simulink model "V2x_TC_IntelligentTrafficSigns_cs"  (Simulink 7.6)
settings: Solver=FixedStepDiscrete  SolverMode=SingleTasking  StartTime=0.0  StopTime=66  FixedStep=1/100  RelTol=1e-3
model workspace (MAT data in the model file):
  mbox_table : struct (value not decoded)
PreLoadFcn:
  | check_NUTS_defined
  | 
  | assignin('base',['model_closed_' bdroot],false);addpath('../includes_V2x');
InitFcn:
  | PreScanExperimentInitFcn(bdroot)
StopFcn:
  | PreScanExperimentStopFcn(bdroot);
  | 
undefined symbols (not defined in the file or in its folder): acceleration, actorID, actorType, DiscIntNeverNeededParam, GPS_alt, GPS_lat, GPS_long, lightBarInUse, messageID, Newton, publicActorType, Raphson, rotZ, sigbuilder_block, sireneInUse, trafficLightPriority, turnAdvice, velocity, x_ini_VRML, y_ini_VRML, yawRate, z_ini_VRML
libraries: simulink x111, PreScanSimulinkLibrary x7, simulink_extras x4, PreScanUsefulBlocks x3
(part 1 of 16)
CloseFcn:
  | evalin('base',['clear model_closed_' bdroot]);
  | 
  | try
  |     close (V2x_TC_GUI)
  | end
  | 
  | try
  |     close (V2X_PlotSpeed)
  | end
settings Model: Creator=PRESCAN  LastModifiedBy=buildbot
settings OptimizationCC: InitFltsAndDblsToZero=on
settings DebuggingCC: SaveWithParameterizedLinksMsg=none  SignalResolutionControl=TryResolveAllWithWarning  MaxConsecutiveZCsMsg=warning  MultiTaskDSMMsg=warning  MultiTaskCondExecSysMsg=none
settings SFSimCC: SimParseCustomCode=off
settings RTWCC: IncludeERTFirstTime=on
settings GRTTargetCC: TargetFcnLib=ansi_tfl_tmw.mat

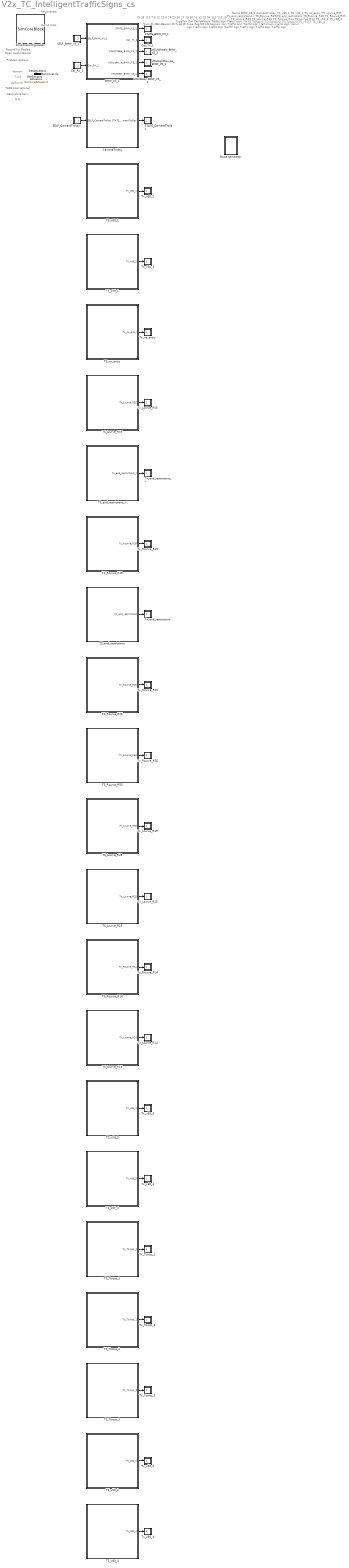
== system V2x_TC_IntelligentTrafficSigns_cs ==
  note: OBU-Beacon ID
  note: Demo Help
  note: Regenerate
  note: Record for Replay
  note: Open Useful Blocks
  note: PreScan Options
  note: Version:
  note: 7.4.0
  note: Author(s):
  note: TASS International
  note: Descriptive text:
  note: N.A.
  note: ID
      |
      | 28
      | 113
      | 7
      | 6
      | 22
      | 23
      | 8
      | 24
      | 25
      | 26
      | 27
      | 29
      | 30
      | 31
      | 32
      | 33
      | 34
      | 111
      | 112
      | 19
      | 20
      | 38
  note: OBU-Beacon ID
  note: TypeID
      |
      | 1
      | 5
      | 14
      | 14
      | 14
      | 14
      | 14
      | 14
      | 14
      | 14
      | 14
      | 14
      | 14
      | 14
      | 14
      | 14
      | 14
      | 14
      | 14
      | 14
      | 14
      | 14
  note: TypeTag
      |
      | Car
      | Cal element
      | Traffic sign
      | Traffic sign
      | Traffic sign
      | Traffic sign
      | Traffic sign
      | Traffic sign
      | Traffic sign
      | Traffic sign
      | Traffic sign
      | Traffic sign
      | Traffic sign
      | Traffic sign
      | Traffic sign
      | Traffic sign
      | Traffic sign
      | Traffic sign
      | Traffic sign
      | Traffic sign
      | Traffic sign
      | Traffic sign
  note: SD
      |
      | Y
      | Y
      | N
      | N
      | N
      | N
      | N
      | N
      | N
      | N
      | N
      | N
      | N
      | N
      | N
      | N
      | N
      | N
      | N
      | N
      | N
      | N
  note: CD
      |
      | N
      | N
      | N
      | N
      | N
      | N
      | N
      | N
      | N
      | N
      | N
      | N
      | N
      | N
      | N
      | N
      | N
      | N
      | N
      | N
      | N
      | N
  note: Name
      |
      | BMW_X5_1
      | CameraTrolley
      | TS_v80_1
      | TS_v50_1
      | TS_no_entry
      | TS_Lcurve_R55
      | TS_end_restrictions_r
      | TS_Rcurve_R39
      | TS_end_restrictions
      | TS_Rcurve_R25
      | TS_Rcurve_R55
      | TS_Lcurve_R39
      | TS_Lcurve_R25
      | TS_Rcurve_R14
      | TS_Lcurve_R14
      | TS_v50_3
      | TS_v80_3
      | TS_Tcross_1
      | TS_Tcross_2
      | TS_Tcross_3
      | TS_v50_2
      | TS_v80_4
  note: Num ID OBU-Beacon ID Type ID Type Tag SD CD Name
  Actuators : DataStoreMemory  Tag=Actuators  DataStoreName=SimCoreActuators  InitialValue=zeros(4563,1)
      settings: DeleteFcn=PreScanBlockDeleteFcn;
  BMW_X5_1 : Subsystem  Tag=WorldObjectSubSystem  InitFcn=UniqueId = '93';
      mask Display: image(imread('BMW_X5.jpg'));color('white');text(0,0,' ID=28\n')
  CameraTrolley : Subsystem  Tag=WorldObjectSubSystem  InitFcn=UniqueId = '98';
      mask Display: image(imread('Sphere.jpg'));color('white');text(0,0,' ID=113\n')
  Car_Rx_1 : Subsystem  Tag=AntennaR  Priority=3  mask(PreScan Source Block: Antenna Receiver){Channel=1, NrEntitiesOut=5, PortName=Car_Rx_1, PortIndex=8, PortWidth=1140, TimeStep=1/100}
      mask prompts: Channel "User Specified: Channel# [-]"; NrEntitiesOut "User Specified: # of Visible Actors"; PortName "Auto Assigned: Port Name [-]"; PortIndex "Auto Assigned: Index [-]"; PortWidth "Auto Assigned: Port Dimensions [-]"; TimeStep "Auto Assigned: Upload Period [s]"
      mask help:
      | Using the Antenna Receiver (RX) and Transmitter (TX) information between actors can be exchanged. This is done over a channel number to be specified by the user.
      |
      | The Antenna Reciever receives actor information and user-definable signals.
      |
      | For more information, please click Help.
      settings: DeleteFcn=PreScanBlockDeleteFcn;
      mask Help: eval('openHelp(424)')
      mask Display: image(imread('Rx.png'))
  Car_Tx_1 : Subsystem  Tag=AntennaT  Priority=0  mask(PreScan Sink Block: AntennaT){Channel=1, PortName=Car_Tx_1, PortIndex=15, PortWidth=205, TimeStep=1/100}
      mask prompts: Channel "User Specified: Channel# [-]"; PortName "Auto Assigned: Port Name [-]"; PortIndex "Auto Assigned: Index [-]"; PortWidth "Auto Assigned: Port Dimensions [-]"; TimeStep "Auto Assigned: Upload Period [s]"
      mask help:
      | Using the Antenna Receiver (RX) and Transmitter (TX) information between actors can be exchanged. This is done over a channel number to be specified by the user.
      |
      | The Antenna Transmitter contains the following, user-definable signals:
      |
      | - Warning Signal ID	[-]
      | - Maneuvre ID		[-]
      | - Vehicle Type 		[-]
      | - Braking Capacity	[-]
      | - Vehicle Size		[-]
      | - Spares (1:10)		[-]
      |
      | For more information, please click Help.
      settings: DeleteFcn=PreScanBlockDeleteFcn;
      mask Help: eval('openHelp(424)')
      mask Display: image(imread('Tx.png'))
  GetSensors : Subsystem  Tag=GetSensors  Priority=2  mask(PreScan Simulation Core Block){NrOfSensors=1156, TimeStep=1/100}
      mask prompts: NrOfSensors "Number of sensor values"; TimeStep "Timestep [s]"
      mask help:
      | Please do not remove this block from the PreScan compilation sheet,
      | as it contains essential configuration parameters for a PreScan simulation.
      settings: DeleteFcn=PreScanBlockDeleteFcn;
  Noise generator : Subsystem
  SELF_BMW_X5_1 : Subsystem  Tag=SELF  Priority=3  mask(PreScan Source Block: SELF){PortName=SELF_BMW_X5_1, PortIndex=0, PortWidth=8, TimeStep=1/100}
      mask prompts: PortName "Auto-Assigned: Port Name [-]"; PortIndex "Auto-Assigned: Port Index [-]"; PortWidth "Auto-Assigned: Port Dimensions"; TimeStep "Auto-Assigned: Upload Period [s]"
      mask help:
      | The SELF port shows (state) information of the actor itself. The outputs are position in the experiment's axis system and the GPS coordinates, and the object's absolute velocity and compass heading.
      |
      | For more information, please click Help.
      settings: DeleteFcn=PreScanBlockDeleteFcn;
      mask Help: eval('openHelp(462)')
      mask Display: image(imread('GPS.png'))
  SELF_CameraTrolley : Subsystem  Tag=SELF  Priority=3  mask(PreScan Source Block: SELF){PortName=SELF_CameraTrolley, PortIndex=1148, PortWidth=8, TimeStep=1/100}
      mask prompts: PortName "Auto-Assigned: Port Name [-]"; PortIndex "Auto-Assigned: Port Index [-]"; PortWidth "Auto-Assigned: Port Dimensions"; TimeStep "Auto-Assigned: Upload Period [s]"
      mask help:
      | The SELF port shows (state) information of the actor itself. The outputs are position in the experiment's axis system and the GPS coordinates, and the object's absolute velocity and compass heading.
      |
      | For more information, please click Help.
      settings: DeleteFcn=PreScanBlockDeleteFcn;
      mask Help: eval('openHelp(462)')
      mask Display: image(imread('GPS.png'))
  STATE_BMW_X5_1 : Subsystem  Tag=State  Priority=0  mask(PreScan Sink Block: State){x0=80, y0=1.75, z0=0.69, Roll0=0, Pitch0=0, Yaw0=179.999588, TimeStep=1/100}
      mask prompts: x0 "Ini x-position VEHICLE wrt GLOBAL axis system [m]"; y0 "Ini y-position VEHICLE wrt GLOBAL axis system [m]"; z0 "Ini z-position VEHICLE wrt GLOBAL axis system [m]"; Roll0 "Ini roll-angle BODY wrt VEHICLE axis system [deg]"; Pitch0 "Ini pitch-angle BODY wrt VEHICLE axis system [deg]"; Yaw0 "Ini yaw-angle VEHICLE wrt GLOBAL axis system [deg]"; TimeStep "Time Step [s]"
      mask help:
      | The Actor State contains a vector signal of size (9x1), containing the following signals:
      |
      | -  Position                  (x,y,z)                                     [m]
      | -  Velocity                  (vx,vy,vz)                               [m/s]
      | -  Angles                    (Roll, Pitch, Yaw)                   [rad]
      |
      | The Actor state is defined in the global axis system.
      | The state is superponed on the Initial position defined in the GUI.
      |
      | For more information, please click Help.
      settings: DeleteFcn=PreScanBlockDeleteFcn;
      mask Help: eval('openHelp(222)')
      mask Display: image(imread('State.png'))
  STATE_CameraTrolley : Subsystem  Tag=State  Priority=0  mask(PreScan Sink Block: State){x0=80, y0=1.75, z0=0.69, Roll0=0, Pitch0=0, Yaw0=179.9996, TimeStep=1/100}
      mask prompts: x0 "Ini x-position VEHICLE wrt GLOBAL axis system [m]"; y0 "Ini y-position VEHICLE wrt GLOBAL axis system [m]"; z0 "Ini z-position VEHICLE wrt GLOBAL axis system [m]"; Roll0 "Ini roll-angle BODY wrt VEHICLE axis system [deg]"; Pitch0 "Ini pitch-angle BODY wrt VEHICLE axis system [deg]"; Yaw0 "Ini yaw-angle VEHICLE wrt GLOBAL axis system [deg]"; TimeStep "Time Step [s]"
      mask help:
      | The Actor State contains a vector signal of size (9x1), containing the following signals:
      |
      | -  Position                  (x,y,z)                                     [m]
      | -  Velocity                  (vx,vy,vz)                               [m/s]
      | -  Angles                    (Roll, Pitch, Yaw)                   [rad]
      |
      | The Actor state is defined in the global axis system.
      | The state is superponed on the Initial position defined in the GUI.
      |
      | For more information, please click Help.
      settings: DeleteFcn=PreScanBlockDeleteFcn;
      mask Help: eval('openHelp(222)')
      mask Display: image(imread('State.png'))
  SetActuators : Subsystem  Tag=SetActuators  Priority=1  mask(PreScan Simulation Core Block){TimeStep=1/100, NrOfActuators=4563}
      mask prompts: TimeStep "Timestep [s]"; NrOfActuators "Number of actuator values"
      mask help:
      | Please do not remove this block from the PreScan compilation sheet,
      | as it contains essential configuration parameters for a PreScan simulation.
      settings: DeleteFcn=PreScanBlockDeleteFcn;
  SimCoreConfiguration : M-S-Function  Tag=SimCoreConfiguration  Description=AE5066EEE462D865D5F999FC1C49C4DE43F133A736DE7B82F5B9C11F164EF63C  FunctionName=SimCoreBlock  Parameters=ExperimentXML PortsXML SimulationCore HostName CheckStart EnableLog RfR EnableGPUAccel ShowComputationTime  mask(PreScan Simulation Core Configurator){SimulationCore=NUTS, HostName=0, CheckStart=0, prescanversion='7.4.0', EnableLog=off, RfR=0, EnableGPUAccel=off, ShowComputationTime=off}
      mask prompts: SimulationCore "Simulation Core"; HostName "Simulation Host"; CheckStart "Show START Dialog"; prescanversion "PreScan Version"; EnableLog "Create TA Log"; RfR "Record for Replay"; EnableGPUAccel "Enable GPU Acceleration"; ShowComputationTime "Show ComputationTime Scope"
      mask help:
      | Please do not remove this block from the PreScan compilation sheet,
      | as it contains essential configuration parameter for a PreScan simulation.
      mask init:
      | model = bdroot;
      | ExperimentXML = [model(1:end-3) '.pex'];
      | PortsXML = [model '_ports.pcd'];
      settings: DeleteFcn=PreScanBlockDeleteFcn;  ModelCloseFcn=assignin('base',['model_closed_' bdroot],true);
      mask Help: eval('openHelp(812)')
      mask Display: image(imread('prescanlogo.ico'))
  SimCoreInfo : Subsystem  Tag=SimCoreInfo
  TS_Lcurve_R14 : Subsystem  Tag=WorldObjectSubSystem  InitFcn=UniqueId = '76';
      mask Display: image(imread('TrafficSignPoleNL.jpg'));color('white');text(0,0,' ID=32\n')
  TS_Lcurve_R25 : Subsystem  Tag=WorldObjectSubSystem  InitFcn=UniqueId = '72';
      mask Display: image(imread('TrafficSignPoleNL.jpg'));color('white');text(0,0,' ID=30\n')
  TS_Lcurve_R39 : Subsystem  Tag=WorldObjectSubSystem  InitFcn=UniqueId = '70';
      mask Display: image(imread('TrafficSignPoleNL.jpg'));color('white');text(0,0,' ID=29\n')
  TS_Lcurve_R55 : Subsystem  Tag=WorldObjectSubSystem  InitFcn=UniqueId = '58';
      mask Display: image(imread('TrafficSignPoleNL.jpg'));color('white');text(0,0,' ID=23\n')
  TS_Rcurve_R14 : Subsystem  Tag=WorldObjectSubSystem  InitFcn=UniqueId = '74';
      mask Display: image(imread('TrafficSignPoleNL.jpg'));color('white');text(0,0,' ID=31\n')
  TS_Rcurve_R25 : Subsystem  Tag=WorldObjectSubSystem  InitFcn=UniqueId = '66';
      mask Display: image(imread('TrafficSignPoleNL.jpg'));color('white');text(0,0,' ID=26\n')
  TS_Rcurve_R39 : Subsystem  Tag=WorldObjectSubSystem  InitFcn=UniqueId = '62';
      mask Display: image(imread('TrafficSignPoleNL.jpg'));color('white');text(0,0,' ID=24\n')
  TS_Rcurve_R55 : Subsystem  Tag=WorldObjectSubSystem  InitFcn=UniqueId = '68';
      mask Display: image(imread('TrafficSignPoleNL.jpg'));color('white');text(0,0,' ID=27\n')
  TS_Tcross_1 : Subsystem  Tag=WorldObjectSubSystem  InitFcn=UniqueId = '82';
      mask Display: image(imread('TrafficSignPoleNL.jpg'));color('white');text(0,0,' ID=111\n')
  TS_Tcross_2 : Subsystem  Tag=WorldObjectSubSystem  InitFcn=UniqueId = '84';
      mask Display: image(imread('TrafficSignPoleNL.jpg'));color('white');text(0,0,' ID=112\n')
  TS_Tcross_3 : Subsystem  Tag=WorldObjectSubSystem  InitFcn=UniqueId = '120';
      mask Display: image(imread('TrafficSignPoleNL.jpg'));color('white');text(0,0,' ID=19\n')
  TS_end_restrictions : Subsystem  Tag=WorldObjectSubSystem  InitFcn=UniqueId = '64';
      mask Display: image(imread('TrafficSignPoleNL.jpg'));color('white');text(0,0,' ID=25\n')
  TS_end_restrictions_r : Subsystem  Tag=WorldObjectSubSystem  InitFcn=UniqueId = '60';
      mask Display: image(imread('TrafficSignPoleNL.jpg'));color('white');text(0,0,' ID=8\n')
  TS_no_entry : Subsystem  Tag=WorldObjectSubSystem  InitFcn=UniqueId = '56';
      mask Display: image(imread('TrafficSignPoleNL.jpg'));color('white');text(0,0,' ID=22\n')
  TS_v50_1 : Subsystem  Tag=WorldObjectSubSystem  InitFcn=UniqueId = '54';
      mask Display: image(imread('TrafficSignPoleNL.jpg'));color('white');text(0,0,' ID=6\n')
  TS_v50_2 : Subsystem  Tag=WorldObjectSubSystem  InitFcn=UniqueId = '122';
      mask Display: image(imread('TrafficSignPoleNL.jpg'));color('white');text(0,0,' ID=20\n')
  TS_v50_3 : Subsystem  Tag=WorldObjectSubSystem  InitFcn=UniqueId = '78';
      mask Display: image(imread('TrafficSignPoleNL.jpg'));color('white');text(0,0,' ID=33\n')
  TS_v80_1 : Subsystem  Tag=WorldObjectSubSystem  InitFcn=UniqueId = '52';
      mask Display: image(imread('TrafficSignPoleNL.jpg'));color('white');text(0,0,' ID=7\n')
  TS_v80_3 : Subsystem  Tag=WorldObjectSubSystem  InitFcn=UniqueId = '80';
      mask Display: image(imread('TrafficSignPoleNL.jpg'));color('white');text(0,0,' ID=34\n')
  TS_v80_4 : Subsystem  Tag=WorldObjectSubSystem  InitFcn=UniqueId = '125';
      mask Display: image(imread('TrafficSignPoleNL.jpg'));color('white');text(0,0,' ID=38\n')
  Tx_Lcurve_R14 : Subsystem  Tag=AntennaT  Priority=0  mask(PreScan Sink Block: AntennaT){Channel=1, PortName=Tx_Lcurve_R14, PortIndex=2923, PortWidth=205, TimeStep=1/100}
      mask prompts: Channel "User Specified: Channel# [-]"; PortName "Auto Assigned: Port Name [-]"; PortIndex "Auto Assigned: Index [-]"; PortWidth "Auto Assigned: Port Dimensions [-]"; TimeStep "Auto Assigned: Upload Period [s]"
      mask help:
      | Using the Antenna Receiver (RX) and Transmitter (TX) information between actors can be exchanged. This is done over a channel number to be specified by the user.
      |
      | The Antenna Transmitter contains the following, user-definable signals:
      |
      | - Warning Signal ID	[-]
      | - Maneuvre ID		[-]
      | - Vehicle Type 		[-]
      | - Braking Capacity	[-]
      | - Vehicle Size		[-]
      | - Spares (1:10)		[-]
      |
      | For more information, please click Help.
      settings: DeleteFcn=PreScanBlockDeleteFcn;
      mask Help: eval('openHelp(424)')
      mask Display: image(imread('Tx.png'))
  Tx_Lcurve_R25 : Subsystem  Tag=AntennaT  Priority=0  mask(PreScan Sink Block: AntennaT){Channel=1, PortName=Tx_Lcurve_R25, PortIndex=2513, PortWidth=205, TimeStep=1/100}
      mask prompts: Channel "User Specified: Channel# [-]"; PortName "Auto Assigned: Port Name [-]"; PortIndex "Auto Assigned: Index [-]"; PortWidth "Auto Assigned: Port Dimensions [-]"; TimeStep "Auto Assigned: Upload Period [s]"
      mask help:
      | Using the Antenna Receiver (RX) and Transmitter (TX) information between actors can be exchanged. This is done over a channel number to be specified by the user.
      |
      | The Antenna Transmitter contains the following, user-definable signals:
      |
      | - Warning Signal ID	[-]
      | - Maneuvre ID		[-]
      | - Vehicle Type 		[-]
      | - Braking Capacity	[-]
      | - Vehicle Size		[-]
      | - Spares (1:10)		[-]
      |
      | For more information, please click Help.
      settings: DeleteFcn=PreScanBlockDeleteFcn;
      mask Help: eval('openHelp(424)')
      mask Display: image(imread('Tx.png'))
  Tx_Lcurve_R39 : Subsystem  Tag=AntennaT  Priority=0  mask(PreScan Sink Block: AntennaT){Channel=1, PortName=Tx_Lcurve_R39, PortIndex=2308, PortWidth=205, TimeStep=1/100}
      mask prompts: Channel "User Specified: Channel# [-]"; PortName "Auto Assigned: Port Name [-]"; PortIndex "Auto Assigned: Index [-]"; PortWidth "Auto Assigned: Port Dimensions [-]"; TimeStep "Auto Assigned: Upload Period [s]"
      mask help:
      | Using the Antenna Receiver (RX) and Transmitter (TX) information between actors can be exchanged. This is done over a channel number to be specified by the user.
      |
      | The Antenna Transmitter contains the following, user-definable signals:
      |
      | - Warning Signal ID	[-]
      | - Maneuvre ID		[-]
      | - Vehicle Type 		[-]
      | - Braking Capacity	[-]
      | - Vehicle Size		[-]
      | - Spares (1:10)		[-]
      |
      | For more information, please click Help.
      settings: DeleteFcn=PreScanBlockDeleteFcn;
      mask Help: eval('openHelp(424)')
      mask Display: image(imread('Tx.png'))
  Tx_Lcurve_R55 : Subsystem  Tag=AntennaT  Priority=0  mask(PreScan Sink Block: AntennaT){Channel=1, PortName=Tx_Lcurve_R55, PortIndex=1078, PortWidth=205, TimeStep=1/100}
      mask prompts: Channel "User Specified: Channel# [-]"; PortName "Auto Assigned: Port Name [-]"; PortIndex "Auto Assigned: Index [-]"; PortWidth "Auto Assigned: Port Dimensions [-]"; TimeStep "Auto Assigned: Upload Period [s]"
      mask help:
      | Using the Antenna Receiver (RX) and Transmitter (TX) information between actors can be exchanged. This is done over a channel number to be specified by the user.
      |
      | The Antenna Transmitter contains the following, user-definable signals:
      |
      | - Warning Signal ID	[-]
      | - Maneuvre ID		[-]
      | - Vehicle Type 		[-]
      | - Braking Capacity	[-]
      | - Vehicle Size		[-]
      | - Spares (1:10)		[-]
      |
      | For more information, please click Help.
      settings: DeleteFcn=PreScanBlockDeleteFcn;
      mask Help: eval('openHelp(424)')
      mask Display: image(imread('Tx.png'))
  Tx_Rcurve_R14 : Subsystem  Tag=AntennaT  Priority=0  mask(PreScan Sink Block: AntennaT){Channel=1, PortName=Tx_Rcurve_R14, PortIndex=2718, PortWidth=205, TimeStep=1/100}
      mask prompts: Channel "User Specified: Channel# [-]"; PortName "Auto Assigned: Port Name [-]"; PortIndex "Auto Assigned: Index [-]"; PortWidth "Auto Assigned: Port Dimensions [-]"; TimeStep "Auto Assigned: Upload Period [s]"
      mask help:
      | Using the Antenna Receiver (RX) and Transmitter (TX) information between actors can be exchanged. This is done over a channel number to be specified by the user.
      |
      | The Antenna Transmitter contains the following, user-definable signals:
      |
      | - Warning Signal ID	[-]
      | - Maneuvre ID		[-]
      | - Vehicle Type 		[-]
      | - Braking Capacity	[-]
      | - Vehicle Size		[-]
      | - Spares (1:10)		[-]
      |
      | For more information, please click Help.
      settings: DeleteFcn=PreScanBlockDeleteFcn;
      mask Help: eval('openHelp(424)')
      mask Display: image(imread('Tx.png'))
  Tx_Rcurve_R25 : Subsystem  Tag=AntennaT  Priority=0  mask(PreScan Sink Block: AntennaT){Channel=1, PortName=Tx_Rcurve_R25, PortIndex=1898, PortWidth=205, TimeStep=1/100}
      mask prompts: Channel "User Specified: Channel# [-]"; PortName "Auto Assigned: Port Name [-]"; PortIndex "Auto Assigned: Index [-]"; PortWidth "Auto Assigned: Port Dimensions [-]"; TimeStep "Auto Assigned: Upload Period [s]"
      mask help:
      | Using the Antenna Receiver (RX) and Transmitter (TX) information between actors can be exchanged. This is done over a channel number to be specified by the user.
      |
      | The Antenna Transmitter contains the following, user-definable signals:
      |
      | - Warning Signal ID	[-]
      | - Maneuvre ID		[-]
      | - Vehicle Type 		[-]
      | - Braking Capacity	[-]
      | - Vehicle Size		[-]
      | - Spares (1:10)		[-]
      |
      | For more information, please click Help.
      settings: DeleteFcn=PreScanBlockDeleteFcn;
      mask Help: eval('openHelp(424)')
      mask Display: image(imread('Tx.png'))
  Tx_Rcurve_R39 : Subsystem  Tag=AntennaT  Priority=0  mask(PreScan Sink Block: AntennaT){Channel=1, PortName=Tx_Rcurve_R39, PortIndex=1488, PortWidth=205, TimeStep=1/100}
      mask prompts: Channel "User Specified: Channel# [-]"; PortName "Auto Assigned: Port Name [-]"; PortIndex "Auto Assigned: Index [-]"; PortWidth "Auto Assigned: Port Dimensions [-]"; TimeStep "Auto Assigned: Upload Period [s]"
      mask help:
      | Using the Antenna Receiver (RX) and Transmitter (TX) information between actors can be exchanged. This is done over a channel number to be specified by the user.
      |
      | The Antenna Transmitter contains the following, user-definable signals:
      |
      | - Warning Signal ID	[-]
      | - Maneuvre ID		[-]
      | - Vehicle Type 		[-]
      | - Braking Capacity	[-]
      | - Vehicle Size		[-]
      | - Spares (1:10)		[-]
      |
      | For more information, please click Help.
      settings: DeleteFcn=PreScanBlockDeleteFcn;
      mask Help: eval('openHelp(424)')
      mask Display: image(imread('Tx.png'))
  Tx_Rcurve_R55 : Subsystem  Tag=AntennaT  Priority=0  mask(PreScan Sink Block: AntennaT){Channel=1, PortName=Tx_Rcurve_R55, PortIndex=2103, PortWidth=205, TimeStep=1/100}
      mask prompts: Channel "User Specified: Channel# [-]"; PortName "Auto Assigned: Port Name [-]"; PortIndex "Auto Assigned: Index [-]"; PortWidth "Auto Assigned: Port Dimensions [-]"; TimeStep "Auto Assigned: Upload Period [s]"
      mask help:
      | Using the Antenna Receiver (RX) and Transmitter (TX) information between actors can be exchanged. This is done over a channel number to be specified by the user.
      |
      | The Antenna Transmitter contains the following, user-definable signals:
      |
      | - Warning Signal ID	[-]
      | - Maneuvre ID		[-]
      | - Vehicle Type 		[-]
      | - Braking Capacity	[-]
      | - Vehicle Size		[-]
      | - Spares (1:10)		[-]
      |
      | For more information, please click Help.
      settings: DeleteFcn=PreScanBlockDeleteFcn;
      mask Help: eval('openHelp(424)')
      mask Display: image(imread('Tx.png'))
  Tx_Tcross_1 : Subsystem  Tag=AntennaT  Priority=0  mask(PreScan Sink Block: AntennaT){Channel=1, PortName=Tx_Tcross_1, PortIndex=3538, PortWidth=205, TimeStep=1/100}
      mask prompts: Channel "User Specified: Channel# [-]"; PortName "Auto Assigned: Port Name [-]"; PortIndex "Auto Assigned: Index [-]"; PortWidth "Auto Assigned: Port Dimensions [-]"; TimeStep "Auto Assigned: Upload Period [s]"
      mask help:
      | Using the Antenna Receiver (RX) and Transmitter (TX) information between actors can be exchanged. This is done over a channel number to be specified by the user.
      |
      | The Antenna Transmitter contains the following, user-definable signals:
      |
      | - Warning Signal ID	[-]
      | - Maneuvre ID		[-]
      | - Vehicle Type 		[-]
      | - Braking Capacity	[-]
      | - Vehicle Size		[-]
      | - Spares (1:10)		[-]
      |
      | For more information, please click Help.
      settings: DeleteFcn=PreScanBlockDeleteFcn;
      mask Help: eval('openHelp(424)')
      mask Display: image(imread('Tx.png'))
  Tx_Tcross_2 : Subsystem  Tag=AntennaT  Priority=0  mask(PreScan Sink Block: AntennaT){Channel=1, PortName=Tx_Tcross_2, PortIndex=3743, PortWidth=205, TimeStep=1/100}
      mask prompts: Channel "User Specified: Channel# [-]"; PortName "Auto Assigned: Port Name [-]"; PortIndex "Auto Assigned: Index [-]"; PortWidth "Auto Assigned: Port Dimensions [-]"; TimeStep "Auto Assigned: Upload Period [s]"
      mask help:
      | Using the Antenna Receiver (RX) and Transmitter (TX) information between actors can be exchanged. This is done over a channel number to be specified by the user.
      |
      | The Antenna Transmitter contains the following, user-definable signals:
      |
      | - Warning Signal ID	[-]
      | - Maneuvre ID		[-]
      | - Vehicle Type 		[-]
      | - Braking Capacity	[-]
      | - Vehicle Size		[-]
      | - Spares (1:10)		[-]
      |
      | For more information, please click Help.
      settings: DeleteFcn=PreScanBlockDeleteFcn;
      mask Help: eval('openHelp(424)')
      mask Display: image(imread('Tx.png'))
  Tx_Tcross_3 : Subsystem  Tag=AntennaT  Priority=0  mask(PreScan Sink Block: AntennaT){Channel=1, PortName=Tx_Tcross_3, PortIndex=3948, PortWidth=205, TimeStep=1/100}
      mask prompts: Channel "User Specified: Channel# [-]"; PortName "Auto Assigned: Port Name [-]"; PortIndex "Auto Assigned: Index [-]"; PortWidth "Auto Assigned: Port Dimensions [-]"; TimeStep "Auto Assigned: Upload Period [s]"
      mask help:
      | Using the Antenna Receiver (RX) and Transmitter (TX) information between actors can be exchanged. This is done over a channel number to be specified by the user.
      |
      | The Antenna Transmitter contains the following, user-definable signals:
      |
      | - Warning Signal ID	[-]
      | - Maneuvre ID		[-]
      | - Vehicle Type 		[-]
      | - Braking Capacity	[-]
      | - Vehicle Size		[-]
      | - Spares (1:10)		[-]
      |
      | For more information, please click Help.
      settings: DeleteFcn=PreScanBlockDeleteFcn;
      mask Help: eval('openHelp(424)')
      mask Display: image(imread('Tx.png'))
  Tx_end_restrictions : Subsystem  Tag=AntennaT  Priority=0  mask(PreScan Sink Block: AntennaT){Channel=1, PortName=Tx_end_restrictions, PortIndex=1693, PortWidth=205, TimeStep=1/100}
      mask prompts: Channel "User Specified: Channel# [-]"; PortName "Auto Assigned: Port Name [-]"; PortIndex "Auto Assigned: Index [-]"; PortWidth "Auto Assigned: Port Dimensions [-]"; TimeStep "Auto Assigned: Upload Period [s]"
      mask help:
      | Using the Antenna Receiver (RX) and Transmitter (TX) information between actors can be exchanged. This is done over a channel number to be specified by the user.
      |
      | The Antenna Transmitter contains the following, user-definable signals:
      |
      | - Warning Signal ID	[-]
      | - Maneuvre ID		[-]
      | - Vehicle Type 		[-]
      | - Braking Capacity	[-]
      | - Vehicle Size		[-]
      | - Spares (1:10)		[-]
      |
      | For more information, please click Help.
      settings: DeleteFcn=PreScanBlockDeleteFcn;
      mask Help: eval('openHelp(424)')
      mask Display: image(imread('Tx.png'))
  Tx_end_restrictions_r : Subsystem  Tag=AntennaT  Priority=0  mask(PreScan Sink Block: AntennaT){Channel=1, PortName=Tx_end_restrictions_r, PortIndex=1283, PortWidth=205, TimeStep=1/100}
      mask prompts: Channel "User Specified: Channel# [-]"; PortName "Auto Assigned: Port Name [-]"; PortIndex "Auto Assigned: Index [-]"; PortWidth "Auto Assigned: Port Dimensions [-]"; TimeStep "Auto Assigned: Upload Period [s]"
      mask help:
      | Using the Antenna Receiver (RX) and Transmitter (TX) information between actors can be exchanged. This is done over a channel number to be specified by the user.
      |
      | The Antenna Transmitter contains the following, user-definable signals:
      |
      | - Warning Signal ID	[-]
      | - Maneuvre ID		[-]
      | - Vehicle Type 		[-]
      | - Braking Capacity	[-]
      | - Vehicle Size		[-]
      | - Spares (1:10)		[-]
      |
      | For more information, please click Help.
      settings: DeleteFcn=PreScanBlockDeleteFcn;
      mask Help: eval('openHelp(424)')
      mask Display: image(imread('Tx.png'))
  Tx_no_entry : Subsystem  Tag=AntennaT  Priority=0  mask(PreScan Sink Block: AntennaT){Channel=1, PortName=Tx_no_entry, PortIndex=873, PortWidth=205, TimeStep=1/100}
      mask prompts: Channel "User Specified: Channel# [-]"; PortName "Auto Assigned: Port Name [-]"; PortIndex "Auto Assigned: Index [-]"; PortWidth "Auto Assigned: Port Dimensions [-]"; TimeStep "Auto Assigned: Upload Period [s]"
      mask help:
      | Using the Antenna Receiver (RX) and Transmitter (TX) information between actors can be exchanged. This is done over a channel number to be specified by the user.
      |
      | The Antenna Transmitter contains the following, user-definable signals:
      |
      | - Warning Signal ID	[-]
      | - Maneuvre ID		[-]
      | - Vehicle Type 		[-]
      | - Braking Capacity	[-]
      | - Vehicle Size		[-]
      | - Spares (1:10)		[-]
      |
      | For more information, please click Help.
      settings: DeleteFcn=PreScanBlockDeleteFcn;
      mask Help: eval('openHelp(424)')
      mask Display: image(imread('Tx.png'))
  Tx_v50_1 : Subsystem  Tag=AntennaT  Priority=0  mask(PreScan Sink Block: AntennaT){Channel=1, PortName=Tx_v50_1, PortIndex=668, PortWidth=205, TimeStep=1/100}
      mask prompts: Channel "User Specified: Channel# [-]"; PortName "Auto Assigned: Port Name [-]"; PortIndex "Auto Assigned: Index [-]"; PortWidth "Auto Assigned: Port Dimensions [-]"; TimeStep "Auto Assigned: Upload Period [s]"
      mask help:
      | Using the Antenna Receiver (RX) and Transmitter (TX) information between actors can be exchanged. This is done over a channel number to be specified by the user.
      |
      | The Antenna Transmitter contains the following, user-definable signals:
      |
      | - Warning Signal ID	[-]
      | - Maneuvre ID		[-]
      | - Vehicle Type 		[-]
      | - Braking Capacity	[-]
      | - Vehicle Size		[-]
      | - Spares (1:10)		[-]
      |
      | For more information, please click Help.
      settings: DeleteFcn=PreScanBlockDeleteFcn;
      mask Help: eval('openHelp(424)')
      mask Display: image(imread('Tx.png'))
  Tx_v50_2 : Subsystem  Tag=AntennaT  Priority=0  mask(PreScan Sink Block: AntennaT){Channel=1, PortName=Tx_v50_2, PortIndex=4153, PortWidth=205, TimeStep=1/100}
      mask prompts: Channel "User Specified: Channel# [-]"; PortName "Auto Assigned: Port Name [-]"; PortIndex "Auto Assigned: Index [-]"; PortWidth "Auto Assigned: Port Dimensions [-]"; TimeStep "Auto Assigned: Upload Period [s]"
      mask help:
      | Using the Antenna Receiver (RX) and Transmitter (TX) information between actors can be exchanged. This is done over a channel number to be specified by the user.
      |
      | The Antenna Transmitter contains the following, user-definable signals:
      |
      | - Warning Signal ID	[-]
      | - Maneuvre ID		[-]
      | - Vehicle Type 		[-]
      | - Braking Capacity	[-]
      | - Vehicle Size		[-]
      | - Spares (1:10)		[-]
      |
      | For more information, please click Help.
      settings: DeleteFcn=PreScanBlockDeleteFcn;
      mask Help: eval('openHelp(424)')
      mask Display: image(imread('Tx.png'))
  Tx_v50_3 : Subsystem  Tag=AntennaT  Priority=0  mask(PreScan Sink Block: AntennaT){Channel=1, PortName=Tx_v50_3, PortIndex=3128, PortWidth=205, TimeStep=1/100}
      mask prompts: Channel "User Specified: Channel# [-]"; PortName "Auto Assigned: Port Name [-]"; PortIndex "Auto Assigned: Index [-]"; PortWidth "Auto Assigned: Port Dimensions [-]"; TimeStep "Auto Assigned: Upload Period [s]"
      mask help:
      | Using the Antenna Receiver (RX) and Transmitter (TX) information between actors can be exchanged. This is done over a channel number to be specified by the user.
      |
      | The Antenna Transmitter contains the following, user-definable signals:
      |
      | - Warning Signal ID	[-]
      | - Maneuvre ID		[-]
      | - Vehicle Type 		[-]
      | - Braking Capacity	[-]
      | - Vehicle Size		[-]
      | - Spares (1:10)		[-]
      |
      | For more information, please click Help.
      settings: DeleteFcn=PreScanBlockDeleteFcn;
      mask Help: eval('openHelp(424)')
      mask Display: image(imread('Tx.png'))
  Tx_v80_1 : Subsystem  Tag=AntennaT  Priority=0  mask(PreScan Sink Block: AntennaT){Channel=1, PortName=Tx_v80_1, PortIndex=463, PortWidth=205, TimeStep=1/100}
      mask prompts: Channel "User Specified: Channel# [-]"; PortName "Auto Assigned: Port Name [-]"; PortIndex "Auto Assigned: Index [-]"; PortWidth "Auto Assigned: Port Dimensions [-]"; TimeStep "Auto Assigned: Upload Period [s]"
      mask help:
      | Using the Antenna Receiver (RX) and Transmitter (TX) information between actors can be exchanged. This is done over a channel number to be specified by the user.
      |
      | The Antenna Transmitter contains the following, user-definable signals:
      |
      | - Warning Signal ID	[-]
      | - Maneuvre ID		[-]
      | - Vehicle Type 		[-]
      | - Braking Capacity	[-]
      | - Vehicle Size		[-]
      | - Spares (1:10)		[-]
      |
      | For more information, please click Help.
      settings: DeleteFcn=PreScanBlockDeleteFcn;
      mask Help: eval('openHelp(424)')
      mask Display: image(imread('Tx.png'))
  Tx_v80_3 : Subsystem  Tag=AntennaT  Priority=0  mask(PreScan Sink Block: AntennaT){Channel=1, PortName=Tx_v80_3, PortIndex=3333, PortWidth=205, TimeStep=1/100}
      mask prompts: Channel "User Specified: Channel# [-]"; PortName "Auto Assigned: Port Name [-]"; PortIndex "Auto Assigned: Index [-]"; PortWidth "Auto Assigned: Port Dimensions [-]"; TimeStep "Auto Assigned: Upload Period [s]"
      mask help:
      | Using the Antenna Receiver (RX) and Transmitter (TX) information between actors can be exchanged. This is done over a channel number to be specified by the user.
      |
      | The Antenna Transmitter contains the following, user-definable signals:
      |
      | - Warning Signal ID	[-]
      | - Maneuvre ID		[-]
      | - Vehicle Type 		[-]
      | - Braking Capacity	[-]
      | - Vehicle Size		[-]
      | - Spares (1:10)		[-]
      |
      | For more information, please click Help.
      settings: DeleteFcn=PreScanBlockDeleteFcn;
      mask Help: eval('openHelp(424)')
      mask Display: image(imread('Tx.png'))
  Tx_v80_4 : Subsystem  Tag=AntennaT  Priority=0  mask(PreScan Sink Block: AntennaT){Channel=1, PortName=Tx_v80_4, PortIndex=4358, PortWidth=205, TimeStep=1/100}
      mask prompts: Channel "User Specified: Channel# [-]"; PortName "Auto Assigned: Port Name [-]"; PortIndex "Auto Assigned: Index [-]"; PortWidth "Auto Assigned: Port Dimensions [-]"; TimeStep "Auto Assigned: Upload Period [s]"
      mask help:
      | Using the Antenna Receiver (RX) and Transmitter (TX) information between actors can be exchanged. This is done over a channel number to be specified by the user.
      |
      | The Antenna Transmitter contains the following, user-definable signals:
      |
      | - Warning Signal ID	[-]
      | - Maneuvre ID		[-]
      | - Vehicle Type 		[-]
      | - Braking Capacity	[-]
      | - Vehicle Size		[-]
      | - Spares (1:10)		[-]
      |
      | For more information, please click Help.
      settings: DeleteFcn=PreScanBlockDeleteFcn;
      mask Help: eval('openHelp(424)')
      mask Display: image(imread('Tx.png'))
  VISULightSource_BMW_X5_1 : Subsystem  Tag=VisuLightSource  Priority=0
      settings: DeleteFcn=PreScanBlockDeleteFcn;
      mask Type: PreScan Sink Block: VisuLightSource
      mask Description:
      | The VisuLightSource block provides a way to animate Light Sources of the indicator, braking, fog and main lights of a vehicle.
      | 
      | For more information, please click Help.
      mask Help: eval('openHelp(223)')
      mask Display: image(imread('RearLight.png'))
  VISUSteer_BMW_X5_1 : Subsystem  Tag=VisuSteer  Priority=0
      settings: DeleteFcn=PreScanBlockDeleteFcn;
      mask Type: PreScan Sink Block: VisuSteer
      mask Description:
      | The VisuSteer block provides a way to adapt the orientation of the steering column and steering wheel of a vehicle.
      | 
      | For more information, please click Help.
      mask Help: eval('openHelp(223)')
      mask Display: image(imread('steeringwheel.png'))
  VISUWheels_BMW_X5_1 : Subsystem  Tag=VisuWheels  Priority=0
      settings: DeleteFcn=PreScanBlockDeleteFcn;
      mask Type: PreScan Sink Block: VisuWheels
      mask Description:
      | The VisuWheels block provides a way to animate the wheels of a vehicle, with steering, rotation (because of velocity) and z-displacement.
      | 
      | For more information, please click Help.
      mask Help: eval('openHelp(223)')
      mask Display: image(imread('wheel.png'))
  wires:
  BMW_X5_1/1(STATE_BMW_X5_1) -> STATE_BMW_X5_1/1(State)
  SELF_BMW_X5_1/1(U) -> BMW_X5_1/1(SELF_BMW_X5_1)
  Car_Rx_1/1(U) -> BMW_X5_1/2(Car_Rx_1)
  BMW_X5_1/2(Car_Tx_1) -> Car_Tx_1/1(Y)
  BMW_X5_1/3(VISUWheels_BMW_X5_1) -> VISUWheels_BMW_X5_1/1(Wheels)
  BMW_X5_1/4(VISULightSource_BMW_X5_1) -> VISULightSource_BMW_X5_1/1(Lights)
  BMW_X5_1/5(VISUSteer_BMW_X5_1) -> VISUSteer_BMW_X5_1/1(Steer)
  CameraTrolley/1(STATE_CameraTrolley) -> STATE_CameraTrolley/1(State)
  SELF_CameraTrolley/1(U) -> CameraTrolley/1(SELF_CameraTrolley)
  TS_v80_1/1(Tx_v80_1) -> Tx_v80_1/1(Y)
  TS_v50_1/1(Tx_v50_1) -> Tx_v50_1/1(Y)
  TS_no_entry/1(Tx_no_entry) -> Tx_no_entry/1(Y)
  TS_Lcurve_R55/1(Tx_Lcurve_R55) -> Tx_Lcurve_R55/1(Y)
  TS_end_restrictions_r/1(Tx_end_restrictions_r) -> Tx_end_restrictions_r/1(Y)
  TS_Rcurve_R39/1(Tx_Rcurve_R39) -> Tx_Rcurve_R39/1(Y)
  TS_end_restrictions/1(Tx_end_restrictions) -> Tx_end_restrictions/1(Y)
  TS_Rcurve_R25/1(Tx_Rcurve_R25) -> Tx_Rcurve_R25/1(Y)
  TS_Rcurve_R55/1(Tx_Rcurve_R55) -> Tx_Rcurve_R55/1(Y)
  TS_Lcurve_R39/1(Tx_Lcurve_R39) -> Tx_Lcurve_R39/1(Y)
  TS_Lcurve_R25/1(Tx_Lcurve_R25) -> Tx_Lcurve_R25/1(Y)
  TS_Rcurve_R14/1(Tx_Rcurve_R14) -> Tx_Rcurve_R14/1(Y)
  TS_Lcurve_R14/1(Tx_Lcurve_R14) -> Tx_Lcurve_R14/1(Y)
  TS_v50_3/1(Tx_v50_3) -> Tx_v50_3/1(Y)
  TS_v80_3/1(Tx_v80_3) -> Tx_v80_3/1(Y)
  TS_Tcross_1/1(Tx_Tcross_1) -> Tx_Tcross_1/1(Y)
  TS_Tcross_2/1(Tx_Tcross_2) -> Tx_Tcross_2/1(Y)
  TS_Tcross_3/1(Tx_Tcross_3) -> Tx_Tcross_3/1(Y)
  TS_v50_2/1(Tx_v50_2) -> Tx_v50_2/1(Y)
  TS_v80_4/1(Tx_v80_4) -> Tx_v80_4/1(Y)
  SetActuators/1(dummy) -> GetSensors/1(dummy)

== system V2x_TC_IntelligentTrafficSigns_cs/BMW_X5_1 ==
  SELF_BMW_X5_1 : Inport  Tag=SELFInport
  Car_Rx_1 : Inport  Tag=AntennaRInport  Port=2
  ANIMATION PORTS Tuning : Subsystem
  Car_Rx_1_BusSelector : BusSelector  Tag=BusAntennaR  OutputSignals=warning,messageID,actorID,actorType,publicActorType,lightBarInUse,sireneInUse,velocity,acceleration,yawRate,turnAdvice,trafficLightPriority,GPS_lat,GPS_long,GPS_alt,rotZ
  Car_Rx_1_Demux : Subsystem  Tag=DemuxAntennaR  mask(PreScan Demux Block:){Channel=1}
      settings: DeleteFcn=PreScanBlockDeleteFcn;
      mask Display:
      | fprintf('Channel: %d',Channel)
      | port_label('output', 1, 'Vehicle_ID')
      | port_label('output', 2, 'GPS Pos Lat [deg]')
      | port_label('output', 3, 'GPS Pos Long [deg]')
      | port_label('output', 4, 'GPS Pos Alt [m]')
      | port_label('output', 5, 'Velocity [m/s]')
      | port_label('output', 6, 'Acceleration [m/s2]')
      | port_label('output', 7, 'Heading [deg]')
      | port_label('output', 8, 'Warning signal ID')
      | port_label('output', 9, 'Maneuvre ID')
      | port_label('output', 10, 'Vehicle Type')
      | port_label('output', 11, 'Braking Capacity')
      | port_label('output', 12, 'Vehicle Size')
      | port_label('output', 13, 'Spare')
  Car_Tx_1_BusAssignment : BusAssignment  Tag=BusAntennaT  AssignedSignals=warning,messageID,actorID,actorType,publicActorType,lightBarInUse,sireneInUse,velocity,acceleration,yawRate,turnAdvice,trafficLightPriority,GPS_lat,GPS_long,GPS_alt,rotZ
  Car_Tx_1_Mux : Subsystem  Tag=MuxAntennaT  mask(PreScan Mux Block:){Channel=1, TimeStep=1/100}
      mask prompts: TimeStep "Time Step [s]"
      settings: DeleteFcn=PreScanBlockDeleteFcn;
      mask Display:
      | fprintf('Channel: %d',Channel)
      | port_label('input', 1, 'Warning signal ID')
      | port_label('input', 2, 'Maneuvre ID')
      | port_label('input', 3, 'Vehicle Type')
      | port_label('input', 4, 'Braking Capacity')
      | port_label('input', 5, 'Vehicle Size')
      | port_label('input', 6, 'spare')
  Ground_{1..14,17..18} (16 alike) : Ground
  Mux : Mux  Inputs=3
  RA_distance : Scope
      settings: YMin=2.5  YMax=55  SaveName=ScopeData7
  RA_warning : Scope
      settings: YMin=-1  YMax=1  SaveName=ScopeData6
  Rate Transition : RateTransition  Integrity=off
  Rx_Noise : Subsystem
  SELF_Demux : Subsystem  Tag=DemuxSELF
      settings: DeleteFcn=PreScanBlockDeleteFcn;
      mask Type: PreScan Demux Block:
      mask Display:
      | port_label('output',1,'x [m]')
      | port_label('output',2,'y [m]')
      | port_label('output',3,'z [m]','texmode','on')
      | port_label('output',4,'Rotx [deg]','texmode','on')
      | port_label('output',5,'Roty [deg]','texmode','on')
      | port_label('output',6,'Rotz [deg]','texmode','on')
      | port_label('output',7,'GPS lat [deg]','texmode','on')
      | port_label('output',8,'GPS long [deg]','texmode','on')
      | port_label('output',9,'GPS alt [m]','texmode','on')
      | port_label('output',10,'Velocity [m/s]')
      | port_label('output',11,'Heading [deg]')
      | port_label('output',12,'Yaw rate [deg/s]')
  Subsystem : Subsystem
  Terminator_{1..20} (20 alike) : Terminator
  Traffic Controller : Subsystem
  Trajectories : Subsystem  Tag=Trajectories
      settings: DeleteFcn=PreScanBlockDeleteFcn;
      mask Type: PreScan Source Block: Trajectories
      mask Description:
      | The Trajectory block contains the trajectories defined in the PreScan Experiment Editor. The output of the trajectory block is the (vehicle) State.
      | 
      | For more information, please click Help.
      mask Help: eval('openHelp(312)')
      mask Display: image(imread('Trajectory.png'));
  Unit Delay : UnitDelay  SampleTime=-1
  V2x_TC_GUI : Subsystem
  VISULightSource_Mux : Subsystem  Tag=MuxLightSource
      settings: DeleteFcn=PreScanBlockDeleteFcn;
      mask Type: PreScan Mux Block:
  VISUSteer_Mux : Subsystem  Tag=MuxSteer
      settings: DeleteFcn=PreScanBlockDeleteFcn;
      mask Type: PreScan Mux Block:
  VISUWheels_Mux : Subsystem  Tag=MuxWheels  mask(PreScan Mux Block:){TimeStep=1/100}
      mask prompts: TimeStep "Auto Assigned: Time Step [s]"
      settings: DeleteFcn=PreScanBlockDeleteFcn;
  hostTurnAdvie : Constant  Value=0
  "intersection warning {left,straight,right}" : Scope
      settings: YMin=-0.1  YMax=1.1  SaveName=ScopeData5
  lightsMessage : Scope
      settings: SaveName=ScopeData2
  lightsPriority : Scope
      settings: SaveName=ScopeData3
  lightsWarning : Scope
      settings: SaveName=ScopeData4
  signsMessage : Scope
      settings: TimeRange=0.1843234764159973  YMin=900.4078101828966  YMax=905.9158957700726
  signsWarning : Scope
  vehicle CAM generator : Subsystem
  STATE_BMW_X5_1 : Outport  Tag=StateOutport
  Car_Tx_1 : Outport  Tag=AntennaTOutport  Port=2
  VISUWheels_BMW_X5_1 : Outport  Tag=VisuWheelsOutport  Port=3  SampleTime=1/100
  VISULightSource_BMW_X5_1 : Outport  Tag=VisuLightsOutport  Port=4  SampleTime=1/100
  VISUSteer_BMW_X5_1 : Outport  Tag=VisuSteerOutport  Port=5  SampleTime=1/100
  tag scope: State_BMW=global
  wires:
  Traffic Controller/2(signsMessage) -> signsMessage/1, V2x_TC_GUI/2(message)
  Traffic Controller/1(signsWarning) -> signsWarning/1, V2x_TC_GUI/1(warning)
  Traffic Controller/3(lightsMessage) -> lightsMessage/1
  Traffic Controller/4(lightsPriority) -> lightsPriority/1
  Traffic Controller/5(lightsWarning) -> lightsWarning/1
  Mux/1 -> "intersection warning {left,straight,right}"/1
  Traffic Controller/6(warning left lane) -> Mux/1
  Traffic Controller/7(warning straight lane) -> Mux/2
  Traffic Controller/8(warning right lane) -> Mux/3
  Traffic Controller/9(RA_warning) -> RA_warning/1
  Traffic Controller/10(RA_distance) -> RA_distance/1
  [State_BMW] -> Rate Transition/1
  hostTurnAdvie/1 -> vehicle CAM generator/10(turnAdvice), Traffic Controller/1(hostTurnAdvice)
  Rx_Noise/13(CAM_GPSlat) -> Traffic Controller/14(GPS_lat)
  Rx_Noise/14(CAM_GPSlong) -> Traffic Controller/15(GPS_long)
  Rx_Noise/15(CAM_GPSalt) -> Traffic Controller/16(GPS_alt)
  Rx_Noise/1(CAM_warning) -> Traffic Controller/7(warning)
  Rx_Noise/2(CAM_messageID) -> Traffic Controller/8(messageID)
  Rx_Noise/3(CAM_vehicleID) -> Traffic Controller/9(actorID)
  Rx_Noise/4(CAM_vehicleType) -> Traffic Controller/10(actorType)
  Rx_Noise/8(CAM_velocity) -> Traffic Controller/11(velocity)
  Rx_Noise/11(CAM_turnAdvice) -> Traffic Controller/12(turnAdvice)
  Rx_Noise/12(CAM_trafficLightPriority) -> Traffic Controller/13(trafficLightPriority)
  Rx_Noise/16(CAM_rotZ) -> Traffic Controller/17(rotZ)
  [NMM_Noise] -> Rx_Noise/17(NoMissedMessages)
  [Tdelay] -> Rx_Noise/18(MessageDelay)
  Rx_Noise/5(CAM_publicVehicleType) -> Terminator_13/1
  Rx_Noise/6(CAM_lightBarInUse) -> Terminator_14/1
  Rx_Noise/7(CAM_sireneInUse) -> Terminator_15/1
  Rx_Noise/9(CAM_acceleration) -> Terminator_16/1
  Rx_Noise/10(CAM_yawRate) -> Terminator_17/1
  Rate Transition/1 -> ANIMATION PORTS Tuning/1(state)
  SELF_BMW_X5_1/1 -> SELF_Demux/1(SELF)
  Car_Rx_1_Demux/13(spare) -> Car_Rx_1_BusSelector/1
  Car_Rx_1/1 -> Car_Rx_1_Demux/1(AntennaR)
  Car_Tx_1_Mux/2(Out2) -> [Car_Tx_1]
  [Car_Tx_1] -> Car_Tx_1_BusAssignment/1
  Car_Tx_1_BusAssignment/1 -> Unit Delay/1
  Car_Tx_1_Mux/1(Out1) -> Car_Tx_1/1
  VISUWheels_Mux/1(Wheels) -> VISUWheels_BMW_X5_1/1
  VISULightSource_Mux/1(Lights) -> VISULightSource_BMW_X5_1/1
  VISUSteer_Mux/1(Steer) -> VISUSteer_BMW_X5_1/1
  Trajectories/1(State) -> STATE_BMW_X5_1/1, [State_BMW]
  Ground_18/1 -> VISULightSource_Mux/12(BrakeLightRight)
  Ground_17/1 -> VISULightSource_Mux/11(BrakeLightLeft)
  SELF_Demux/11(heading [deg]) -> Terminator_20/1
  SELF_Demux/5(roty [deg]) -> Terminator_19/1
  SELF_Demux/4(rotx [deg]) -> Terminator_18/1
  Car_Rx_1_Demux/12(vehicle_size) -> Terminator_12/1
  Car_Rx_1_Demux/11(brakingcapacity) -> Terminator_11/1
  Car_Rx_1_Demux/10(vehicle_type) -> Terminator_10/1
  vehicle CAM generator/16(CAM_rotZ) -> Car_Tx_1_BusAssignment/17
  vehicle CAM generator/15(CAM_GPSalt) -> Car_Tx_1_BusAssignment/16
  vehicle CAM generator/14(CAM_GPSlong) -> Car_Tx_1_BusAssignment/15
  vehicle CAM generator/13(CAM_GPSlat) -> Car_Tx_1_BusAssignment/14
  vehicle CAM generator/12(CAM_trafficLightPriority) -> Car_Tx_1_BusAssignment/13
  vehicle CAM generator/11(CAM_turnAdvice) -> Car_Tx_1_BusAssignment/12
  Car_Rx_1_BusSelector/16 -> Rx_Noise/16(CAM_rotZ_in)  "<rotZ>"
  Car_Rx_1_BusSelector/15 -> Rx_Noise/15(CAM_GPSalt_in)  "<GPS_alt>"
  Car_Rx_1_BusSelector/14 -> Rx_Noise/14(CAM_GPSlong_in)  "<GPS_long>"
  Car_Rx_1_BusSelector/13 -> Rx_Noise/13(CAM_GPSlat_in)  "<GPS_lat>"
  Car_Rx_1_BusSelector/12 -> Rx_Noise/12(CAM_trafficLightPriority_in)  "<trafficLightPriority>"
  Car_Rx_1_BusSelector/11 -> Rx_Noise/11(CAM_turnAdvice_in)  "<turnAdvice>"
  Car_Rx_1_BusSelector/10 -> Rx_Noise/10(CAM_yawRate_in)  "<yawRate>"
  Car_Rx_1_BusSelector/9 -> Rx_Noise/9(CAM_acceleration_in)  "<acceleration>"
  Car_Rx_1_BusSelector/8 -> Rx_Noise/8(CAM_velocity_in)  "<velocity>"
  Car_Rx_1_BusSelector/7 -> Rx_Noise/7(CAM_sireneInUse_in)  "<sireneInUse>"
  Car_Rx_1_BusSelector/6 -> Rx_Noise/6(CAM_lightBarInUse_in)  "<lightBarInUse>"
  Car_Rx_1_BusSelector/5 -> Rx_Noise/5(CAM_publicVehicleType_in)  "<publicActorType>"
  Car_Rx_1_BusSelector/4 -> Rx_Noise/4(CAM_vehicleType_in)  "<actorType>"
  Car_Rx_1_BusSelector/3 -> Rx_Noise/3(CAM_vehicleID_in)  "<actorID>"
  Car_Rx_1_BusSelector/2 -> Rx_Noise/2(CAM_messageID_in)  "<messageID>"
  Car_Rx_1_BusSelector/1 -> Rx_Noise/1(CAM_warning_in)  "<warning>"
  SELF_Demux/2(y [m]) -> vehicle CAM generator/2(y [m])
  SELF_Demux/1(x [m]) -> vehicle CAM generator/1(x [m])
  SELF_Demux/12(yaw rate [deg/s]) -> vehicle CAM generator/9(yawRate)
  SELF_Demux/10(velocity [m/s]) -> vehicle CAM generator/8(velocity [m/s]), Traffic Controller/6(hostVelocity)
  SELF_Demux/9(GPS alt [m]) -> vehicle CAM generator/7(GPS_alt), Traffic Controller/5(host GPS alt [m])
  SELF_Demux/8(GPS long [deg]) -> vehicle CAM generator/6(GPS_long), Traffic Controller/4(host GPS long [deg])
  SELF_Demux/7(GPS lat [deg]) -> vehicle CAM generator/5(GPS_lat), Traffic Controller/3(host GPS lat [deg])
  SELF_Demux/6(rotz [deg]) -> vehicle CAM generator/4(rotZ [deg]), Traffic Controller/2(hostRotZ)
  SELF_Demux/3(z [m]) -> vehicle CAM generator/3(z [m])
  ANIMATION PORTS Tuning/8(Steer [deg]) -> VISUSteer_Mux/2(Steer [deg])
  ANIMATION PORTS Tuning/7(Column [deg]) -> VISUSteer_Mux/1(Column [deg])
  ANIMATION PORTS Tuning/6(zDisp RR [m]) -> VISUWheels_Mux/7(zDisp RR [m])
  ANIMATION PORTS Tuning/5(zDisp RL [m]) -> VISUWheels_Mux/6(zDisp RL [m])
  ANIMATION PORTS Tuning/4(zDisp FR [m]) -> VISUWheels_Mux/5(zDisp FR [m])
  ANIMATION PORTS Tuning/3(zDisp FL [m]) -> VISUWheels_Mux/4(zDisp FL [m])
  ANIMATION PORTS Tuning/2(Velocity [m/s]) -> VISUWheels_Mux/3(Velocity [m/s])
  ANIMATION PORTS Tuning/1(Steer Angle Front [deg]) -> VISUWheels_Mux/1(Steer Angle Front [deg])
  vehicle CAM generator/1(CAM_warning) -> Car_Tx_1_BusAssignment/2
  vehicle CAM generator/2(CAM_messageID) -> Car_Tx_1_BusAssignment/3
  vehicle CAM generator/3(CAM_actorID) -> Car_Tx_1_BusAssignment/4
  vehicle CAM generator/4(CAM_actorType) -> Car_Tx_1_BusAssignment/5
  vehicle CAM generator/5(CAM_publicActorType) -> Car_Tx_1_BusAssignment/6
  vehicle CAM generator/6(CAM_lightBarInUse) -> Car_Tx_1_BusAssignment/7
  vehicle CAM generator/7(CAM_sireneInUse) -> Car_Tx_1_BusAssignment/8
  vehicle CAM generator/8(CAM_velocity) -> Car_Tx_1_BusAssignment/9
  vehicle CAM generator/9(CAM_acceleration) -> Car_Tx_1_BusAssignment/10
  vehicle CAM generator/10(CAM_yawRate) -> Car_Tx_1_BusAssignment/11
  Subsystem/1(Left) -> VISULightSource_Mux/1(IndicatorLightsLeft)
  Subsystem/2(Right) -> VISULightSource_Mux/2(IndicatorLightsRight)
  Unit Delay/1 -> Car_Tx_1_Mux/6(spare)
  Car_Rx_1_Demux/1(Vehicle_ID) -> Terminator_1/1
  Car_Rx_1_Demux/2(GPS_pos_lat_[deg]) -> Terminator_2/1
  Car_Rx_1_Demux/3(GPS_pos_long_[deg]) -> Terminator_3/1
  Car_Rx_1_Demux/4(GPS_pos_alt_[m]) -> Terminator_4/1
  Car_Rx_1_Demux/5(Velocity_[m/s]) -> Terminator_5/1
  Car_Rx_1_Demux/6(Acceleration_[m/s2]) -> Terminator_6/1
  Car_Rx_1_Demux/7(Heading [deg]) -> Terminator_7/1
  Car_Rx_1_Demux/8(warning_signal_id) -> Terminator_8/1
  Car_Rx_1_Demux/9(maneuvre_id) -> Terminator_9/1
  Ground_1/1 -> Car_Tx_1_Mux/1(warning_signal_id)
  Ground_2/1 -> Car_Tx_1_Mux/2(maneuvre_id)
  Ground_3/1 -> Car_Tx_1_Mux/3(vehicle_type)
  Ground_4/1 -> Car_Tx_1_Mux/4(brakingcapacity)
  Ground_5/1 -> Car_Tx_1_Mux/5(vehicle_size)
  Ground_6/1 -> VISULightSource_Mux/3(FogLightRearLeft)
  Ground_7/1 -> VISULightSource_Mux/4(FogLightRearRight)
  Ground_8/1 -> VISULightSource_Mux/5(FogLightFrontLeft)
  Ground_9/1 -> VISULightSource_Mux/6(FogLightFrontRight)
  Ground_10/1 -> VISULightSource_Mux/7(MainLightRearLeft)
  Ground_11/1 -> VISULightSource_Mux/8(MainLightRearRight)
  Ground_12/1 -> VISULightSource_Mux/9(MainLightFrontLeft)
  Ground_13/1 -> VISULightSource_Mux/10(MainLightFrontRight)
  Ground_14/1 -> VISUWheels_Mux/2(Steer Angle Rear [deg])
